simulink model "r2_ratty2_32k_r203"  (R2012b) (continued)
== system r2_ratty2_32k_r203/fft/fft_direct == (continued)
      mask prompts: n_inputs "number inputs"; biplex "Used in biplex FFT"; FFTSize "Size of FFT: (2^? pts)"; Coeffs "Coefficients: (0 to 2^(FFTSize-1)-1)"; StepPeriod "Coefficient Step Period: (2^?)"; coeff_bit_width "Coefficient Bit Width"; input_bit_width "Input Bit Width"; bin_pt_in "Input binary point"; async "asynchronous operation"; add_latency "Add Latency"; mult_latency "Mult Latency"; bram_latency "BRAM Latency"; conv_latency "Convert latency"; quantization "Quantization Behavior"; overflow "Overflow Behavior"; coeffs_bit_limit "Number bits above which to store stage's coefficients in BRAM (2^? bits)"; coeff_sharing "BRAM sharing in coeff storage"; coeff_decimation "Store a fraction of coeff factors where useful"; coeff_generation "Generate coeffs with multipliers where useful"; cal_bits "Number calibration locations when generating coeffs (2^?)"; n_bits_rotation "Feedback rotation vector resolution"; max_fanout "Maximum fanout"; use_hdl "Use behavioural HDL for multipliers"; use_embedded "Use embedded multipliers"; bitgrowth "Bit growth instead of shifting"; hardcode_shifts "Hardcode shift schedule"; dsp48_adders "Use DSP48s for adders"
      mask help: FFT internal block.
      mask init: same as r2_ratty2_32k_r203/fft/fft_direct/butterfly0_0
      settings: AttributesFormatString=twiddle_general
      mask TypeOptions quantization: Truncate | Round  (unbiased: +/- Inf) | Round  (unbiased: Even Values)
      mask TypeOptions overflow: Wrap | Saturate | Error
      mask Callback use_hdl:
      | % make mutually exclusive with use_embedded.
      | 
      | use_hdl = get_param(gcb, 'use_hdl');
      | if strcmp(use_hdl, 'on'),
      |     set_param_state(gcb, 'use_embedded', 'off');
      | else
      |     set_param_state(gcb, 'use_embedded', 'on');
      | end
      mask Callback use_embedded:
      | % make mutually exclusive with use_hdl.
      | 
      | use_hdl = get_param(gcb, 'use_embedded');
      | if strcmp(use_hdl, 'on'),
      |     set_param_state(gcb, 'use_hdl', 'off');
      | else
      |     set_param_state(gcb, 'use_hdl', 'on');
      | end
      mask Callback hardcode_shifts:
      | hardcode_shifts = get_param(gcb, 'hardcode_shifts');
      | set_param_state(gcb, 'downshift', hardcode_shifts);
      mask Callback dsp48_adders: %fft_callback_dsp48_adders(gcb);
  butterfly2_0 : Subsystem  AncestorBlock=casper_library_ffts/butterfly_direct  mask(butterfly_direct){n_inputs=1, biplex=off, FFTSize=16, Coeffs=[ 0 16384 8192 24576 4096 20480 12288 28672 2048 18432 10240 26624 6144 22528 14336 30720 1024 17408 9216 25600 5120 21504 13312 29696 3072 19456 11264 27648 7168 23552 15360 31744 512 16896 8704 25088 4608 20992 12800 29184 2560 18944 10752 27136 6656 23040 14848 31232 1536 17920 9728 26112 5632 22016 13824 30208 3584 19968 11776 28160 7680 24064 15872 32256 256 16640 8448 24832 4352 20736 12544 28928 2304 18688 10496 26880 6400 22784 14592 30976 1280 17664 9472 25856 5376 21760 13568 29952 3328 19712 11520 27904 7424 23808 15616 32000 768 17152 8960 25344 4864 21248 13056 29440 2816 19200 11008 27392 6912 23296 15104 31488 1792 18176 9984 26368 5888 22272 14080 30464 3840 20224 12032 28416 7936 24320 16128 32512 128 16512 8320 24704 4224 20608 12416 28800 2176 18560 10368 26752 6272 22656 14464 30848 1152 17536 9344 25728 5248 21632 13440 29824 3200 19584 11392 27776 7296 23680 15488 31872 640 17024 8832 25216 4736 21120 12928 29312 2688 19072 10880 27264 6784 23168 14976 31360 1664 18048 9856 26240 5760 22144 13952 30336 3712 20096 11904 28288 7808 24192 16000 32384 384 16768 8576 24960 4480 20864 12672 29056 2432 18816 10624 27008 6528 22912 14720 31104 1408 17792 9600 25984 5504 21888 13696 30080 3456 19840 11648 28032 7552 23936 15744 32128 896 17280 9088 25472 4992 21376 13184 29568 2944 19328 11136 27520 7040 23424 15232 31616 1920 18304 10112 26496 6016 22400 14208 30592 3968 20352 12160 28544 8064 24448 16256 32640 64 16448 8256 24640 4160 20544 12352 28736 2112 18496 10304 26688 6208 22592 14400 30784 1088 17472 9280 25664 5184 21568 13376 29760 3136 19520 11328 27712 7232 23616 15424 31808 576 16960 8768 25152 4672 21056 12864 29248 2624 19008 10816 27200 6720 23104 14912 31296 1600 17984 9792 26176 5696 22080 13888 30272 3648 20032 11840 28224 7744 24128 15936 32320 320 16704 8512 24896 4416 20800 12608 28992 2368 18752 10560 26944 6464 22848 14656 31040 1344 17728 9536 25920 5440 21824 13632 30016 3392 19776 11584 27968 7488 23872 15680 32064 832 17216 9024 25408 4928 21312 13120 29504 2880 19264 11072 27456 6976 23360 15168 31552 1856 18240 10048 26432 5952 22336 14144 30528 3904 20288 12096 28480 8000 24384 16192 32576 192 16576 8384 24768 4288 20672 12480 28864 2240 18624 10432 26816 6336 22720 14528 30912 1216 17600 9408 25792 5312 21696 13504 29888 3264 19648 11456 27840 7360 23744 15552 31936 704 17088 8896 25280 4800 21184 12992 29376 2752 19136 10944 27328 6848 23232 15040 31424 1728 18112 9920 26304 5824 22208 14016 30400 3776 20160 11968 28352 7872 24256 16064 32448 448 16832 8640 25024 4544 20928 12736 29120 2496 18880 10688 27072 6592 22976 14784 31168 1472 17856 9664 26048 5568 21952 13760 30144 3520 19904 11712 28096 7616 24000 15808 32192 960 17344 9152 25536 5056 21440 13248 29632 3008 19392 11200 27584 7104 23488 15296 31680 1984 18368 10176 26560 6080 22464 14272 30656 4032 20416 12224 28608 8128 24512 16320 32704 32 16416 8224 24608 4128 20512 12320 28704 2080 18464 10272 26656 6176 22560 14368 30752 1056 17440 9248 25632 5152 21536 13344 29728 3104 19488 11296 27680 7200 23584 15392 31776 544 16928 8736 25120 4640 21024 12832 29216 2592 18976 10784 27168 6688 23072 14880 31264 1568 17952 9760 26144 5664 22048 13856 30240 3616 20000 11808 28192 7712 24096 15904 32288 288 16672 8480 24864 4384 20768 12576 28960 2336 18720 10528 26912 6432 22816 14624 31008 1312 17696 9504 25888 5408 21792 13600 29984 3360 19744 11552 27936 7456 23840 15648 32032 800 17184 8992 25376 4896 21280 13088 29472 2848 19232 11040 27424 6944 23328 15136 31520 1824 18208 10016 26400 5920 22304 14112 30496 3872 20256 12064 28448 7968 24352 16160 32544 160 16544 8352 24736 4256 20640 12448 28832 2208 18592 10400 26784 6304 22688 14496 30880 1184 17568 9376 25760 5280 21664 13472 29856 3232 19616 11424 27808 7328 23712 15520 31904 672 17056 8864 25248 4768 21152 12960 29344 2720 19104 10912 27296 6816 23200 15008 31392 1696 18080 9888 26272 5792 22176 13984 30368 3744 20128 11936 28320 7840 24224 16032 32416 416 16800 8608 ...(+42377 chars)], StepPeriod=0, coeff_bit_width=18, input_bit_width=23, bin_pt_in=21, downshift=off, async=off, add_latency=4, mult_latency=5, bram_latency=12, conv_latency=5, quantization=Round  (unbiased: +/- Inf), overflow=Wrap, coeffs_bit_limit=10, coeff_sharing=off, coeff_decimation=off, coeff_generation=off, cal_bits=cal_bits, n_bits_rotation=n_bits_rotation, max_fanout=max_fanout, use_hdl=off, use_embedded=on, bitgrowth=off, hardcode_shifts=off, dsp48_adders=off}
      mask prompts: n_inputs "number inputs"; biplex "Used in biplex FFT"; FFTSize "Size of FFT: (2^? pts)"; Coeffs "Coefficients: (0 to 2^(FFTSize-1)-1)"; StepPeriod "Coefficient Step Period: (2^?)"; coeff_bit_width "Coefficient Bit Width"; input_bit_width "Input Bit Width"; bin_pt_in "Input binary point"; async "asynchronous operation"; add_latency "Add Latency"; mult_latency "Mult Latency"; bram_latency "BRAM Latency"; conv_latency "Convert latency"; quantization "Quantization Behavior"; overflow "Overflow Behavior"; coeffs_bit_limit "Number bits above which to store stage's coefficients in BRAM (2^? bits)"; coeff_sharing "BRAM sharing in coeff storage"; coeff_decimation "Store a fraction of coeff factors where useful"; coeff_generation "Generate coeffs with multipliers where useful"; cal_bits "Number calibration locations when generating coeffs (2^?)"; n_bits_rotation "Feedback rotation vector resolution"; max_fanout "Maximum fanout"; use_hdl "Use behavioural HDL for multipliers"; use_embedded "Use embedded multipliers"; bitgrowth "Bit growth instead of shifting"; hardcode_shifts "Hardcode shift schedule"; dsp48_adders "Use DSP48s for adders"
      mask help: FFT internal block.
      mask init: same as r2_ratty2_32k_r203/fft/fft_direct/butterfly0_0
      settings: AttributesFormatString=twiddle_general
      mask TypeOptions quantization: Truncate | Round  (unbiased: +/- Inf) | Round  (unbiased: Even Values)
      mask TypeOptions overflow: Wrap | Saturate | Error
      mask Callback use_hdl:
      | % make mutually exclusive with use_embedded.
      | 
      | use_hdl = get_param(gcb, 'use_hdl');
      | if strcmp(use_hdl, 'on'),
      |     set_param_state(gcb, 'use_embedded', 'off');
      | else
      |     set_param_state(gcb, 'use_embedded', 'on');
      | end
      mask Callback use_embedded:
      | % make mutually exclusive with use_hdl.
      | 
      | use_hdl = get_param(gcb, 'use_embedded');
      | if strcmp(use_hdl, 'on'),
      |     set_param_state(gcb, 'use_hdl', 'off');
      | else
      |     set_param_state(gcb, 'use_hdl', 'on');
      | end
      mask Callback hardcode_shifts:
      | hardcode_shifts = get_param(gcb, 'hardcode_shifts');
      | set_param_state(gcb, 'downshift', hardcode_shifts);
      mask Callback dsp48_adders: %fft_callback_dsp48_adders(gcb);
  butterfly2_1 : Subsystem  AncestorBlock=casper_library_ffts/butterfly_direct  mask(butterfly_direct){n_inputs=1, biplex=off, FFTSize=16, Coeffs=[ 1 16385 8193 24577 4097 20481 12289 28673 2049 18433 10241 26625 6145 22529 14337 30721 1025 17409 9217 25601 5121 21505 13313 29697 3073 19457 11265 27649 7169 23553 15361 31745 513 16897 8705 25089 4609 20993 12801 29185 2561 18945 10753 27137 6657 23041 14849 31233 1537 17921 9729 26113 5633 22017 13825 30209 3585 19969 11777 28161 7681 24065 15873 32257 257 16641 8449 24833 4353 20737 12545 28929 2305 18689 10497 26881 6401 22785 14593 30977 1281 17665 9473 25857 5377 21761 13569 29953 3329 19713 11521 27905 7425 23809 15617 32001 769 17153 8961 25345 4865 21249 13057 29441 2817 19201 11009 27393 6913 23297 15105 31489 1793 18177 9985 26369 5889 22273 14081 30465 3841 20225 12033 28417 7937 24321 16129 32513 129 16513 8321 24705 4225 20609 12417 28801 2177 18561 10369 26753 6273 22657 14465 30849 1153 17537 9345 25729 5249 21633 13441 29825 3201 19585 11393 27777 7297 23681 15489 31873 641 17025 8833 25217 4737 21121 12929 29313 2689 19073 10881 27265 6785 23169 14977 31361 1665 18049 9857 26241 5761 22145 13953 30337 3713 20097 11905 28289 7809 24193 16001 32385 385 16769 8577 24961 4481 20865 12673 29057 2433 18817 10625 27009 6529 22913 14721 31105 1409 17793 9601 25985 5505 21889 13697 30081 3457 19841 11649 28033 7553 23937 15745 32129 897 17281 9089 25473 4993 21377 13185 29569 2945 19329 11137 27521 7041 23425 15233 31617 1921 18305 10113 26497 6017 22401 14209 30593 3969 20353 12161 28545 8065 24449 16257 32641 65 16449 8257 24641 4161 20545 12353 28737 2113 18497 10305 26689 6209 22593 14401 30785 1089 17473 9281 25665 5185 21569 13377 29761 3137 19521 11329 27713 7233 23617 15425 31809 577 16961 8769 25153 4673 21057 12865 29249 2625 19009 10817 27201 6721 23105 14913 31297 1601 17985 9793 26177 5697 22081 13889 30273 3649 20033 11841 28225 7745 24129 15937 32321 321 16705 8513 24897 4417 20801 12609 28993 2369 18753 10561 26945 6465 22849 14657 31041 1345 17729 9537 25921 5441 21825 13633 30017 3393 19777 11585 27969 7489 23873 15681 32065 833 17217 9025 25409 4929 21313 13121 29505 2881 19265 11073 27457 6977 23361 15169 31553 1857 18241 10049 26433 5953 22337 14145 30529 3905 20289 12097 28481 8001 24385 16193 32577 193 16577 8385 24769 4289 20673 12481 28865 2241 18625 10433 26817 6337 22721 14529 30913 1217 17601 9409 25793 5313 21697 13505 29889 3265 19649 11457 27841 7361 23745 15553 31937 705 17089 8897 25281 4801 21185 12993 29377 2753 19137 10945 27329 6849 23233 15041 31425 1729 18113 9921 26305 5825 22209 14017 30401 3777 20161 11969 28353 7873 24257 16065 32449 449 16833 8641 25025 4545 20929 12737 29121 2497 18881 10689 27073 6593 22977 14785 31169 1473 17857 9665 26049 5569 21953 13761 30145 3521 19905 11713 28097 7617 24001 15809 32193 961 17345 9153 25537 5057 21441 13249 29633 3009 19393 11201 27585 7105 23489 15297 31681 1985 18369 10177 26561 6081 22465 14273 30657 4033 20417 12225 28609 8129 24513 16321 32705 33 16417 8225 24609 4129 20513 12321 28705 2081 18465 10273 26657 6177 22561 14369 30753 1057 17441 9249 25633 5153 21537 13345 29729 3105 19489 11297 27681 7201 23585 15393 31777 545 16929 8737 25121 4641 21025 12833 29217 2593 18977 10785 27169 6689 23073 14881 31265 1569 17953 9761 26145 5665 22049 13857 30241 3617 20001 11809 28193 7713 24097 15905 32289 289 16673 8481 24865 4385 20769 12577 28961 2337 18721 10529 26913 6433 22817 14625 31009 1313 17697 9505 25889 5409 21793 13601 29985 3361 19745 11553 27937 7457 23841 15649 32033 801 17185 8993 25377 4897 21281 13089 29473 2849 19233 11041 27425 6945 23329 15137 31521 1825 18209 10017 26401 5921 22305 14113 30497 3873 20257 12065 28449 7969 24353 16161 32545 161 16545 8353 24737 4257 20641 12449 28833 2209 18593 10401 26785 6305 22689 14497 30881 1185 17569 9377 25761 5281 21665 13473 29857 3233 19617 11425 27809 7329 23713 15521 31905 673 17057 8865 25249 4769 21153 12961 29345 2721 19105 10913 27297 6817 23201 15009 31393 1697 18081 9889 26273 5793 22177 13985 30369 3745 20129 11937 28321 7841 24225 16033 32417 417 16801 8609 ...(+42377 chars)], StepPeriod=0, coeff_bit_width=18, input_bit_width=23, bin_pt_in=21, downshift=off, async=off, add_latency=4, mult_latency=5, bram_latency=12, conv_latency=5, quantization=Round  (unbiased: +/- Inf), overflow=Wrap, coeffs_bit_limit=10, coeff_sharing=off, coeff_decimation=off, coeff_generation=off, cal_bits=cal_bits, n_bits_rotation=n_bits_rotation, max_fanout=max_fanout, use_hdl=off, use_embedded=on, bitgrowth=off, hardcode_shifts=off, dsp48_adders=off}
      mask prompts: n_inputs "number inputs"; biplex "Used in biplex FFT"; FFTSize "Size of FFT: (2^? pts)"; Coeffs "Coefficients: (0 to 2^(FFTSize-1)-1)"; StepPeriod "Coefficient Step Period: (2^?)"; coeff_bit_width "Coefficient Bit Width"; input_bit_width "Input Bit Width"; bin_pt_in "Input binary point"; async "asynchronous operation"; add_latency "Add Latency"; mult_latency "Mult Latency"; bram_latency "BRAM Latency"; conv_latency "Convert latency"; quantization "Quantization Behavior"; overflow "Overflow Behavior"; coeffs_bit_limit "Number bits above which to store stage's coefficients in BRAM (2^? bits)"; coeff_sharing "BRAM sharing in coeff storage"; coeff_decimation "Store a fraction of coeff factors where useful"; coeff_generation "Generate coeffs with multipliers where useful"; cal_bits "Number calibration locations when generating coeffs (2^?)"; n_bits_rotation "Feedback rotation vector resolution"; max_fanout "Maximum fanout"; use_hdl "Use behavioural HDL for multipliers"; use_embedded "Use embedded multipliers"; bitgrowth "Bit growth instead of shifting"; hardcode_shifts "Hardcode shift schedule"; dsp48_adders "Use DSP48s for adders"
      mask help: FFT internal block.
      mask init: same as r2_ratty2_32k_r203/fft/fft_direct/butterfly0_0
      settings: AttributesFormatString=twiddle_general
      mask TypeOptions quantization: Truncate | Round  (unbiased: +/- Inf) | Round  (unbiased: Even Values)
      mask TypeOptions overflow: Wrap | Saturate | Error
      mask Callback use_hdl:
      | % make mutually exclusive with use_embedded.
      | 
      | use_hdl = get_param(gcb, 'use_hdl');
      | if strcmp(use_hdl, 'on'),
      |     set_param_state(gcb, 'use_embedded', 'off');
      | else
      |     set_param_state(gcb, 'use_embedded', 'on');
      | end
      mask Callback use_embedded:
      | % make mutually exclusive with use_hdl.
      | 
      | use_hdl = get_param(gcb, 'use_embedded');
      | if strcmp(use_hdl, 'on'),
      |     set_param_state(gcb, 'use_hdl', 'off');
      | else
      |     set_param_state(gcb, 'use_hdl', 'on');
      | end
      mask Callback hardcode_shifts:
      | hardcode_shifts = get_param(gcb, 'hardcode_shifts');
      | set_param_state(gcb, 'downshift', hardcode_shifts);
      mask Callback dsp48_adders: %fft_callback_dsp48_adders(gcb);
  butterfly2_2 : Subsystem  AncestorBlock=casper_library_ffts/butterfly_direct  mask(butterfly_direct){n_inputs=1, biplex=off, FFTSize=16, Coeffs=[ 2 16386 8194 24578 4098 20482 12290 28674 2050 18434 10242 26626 6146 22530 14338 30722 1026 17410 9218 25602 5122 21506 13314 29698 3074 19458 11266 27650 7170 23554 15362 31746 514 16898 8706 25090 4610 20994 12802 29186 2562 18946 10754 27138 6658 23042 14850 31234 1538 17922 9730 26114 5634 22018 13826 30210 3586 19970 11778 28162 7682 24066 15874 32258 258 16642 8450 24834 4354 20738 12546 28930 2306 18690 10498 26882 6402 22786 14594 30978 1282 17666 9474 25858 5378 21762 13570 29954 3330 19714 11522 27906 7426 23810 15618 32002 770 17154 8962 25346 4866 21250 13058 29442 2818 19202 11010 27394 6914 23298 15106 31490 1794 18178 9986 26370 5890 22274 14082 30466 3842 20226 12034 28418 7938 24322 16130 32514 130 16514 8322 24706 4226 20610 12418 28802 2178 18562 10370 26754 6274 22658 14466 30850 1154 17538 9346 25730 5250 21634 13442 29826 3202 19586 11394 27778 7298 23682 15490 31874 642 17026 8834 25218 4738 21122 12930 29314 2690 19074 10882 27266 6786 23170 14978 31362 1666 18050 9858 26242 5762 22146 13954 30338 3714 20098 11906 28290 7810 24194 16002 32386 386 16770 8578 24962 4482 20866 12674 29058 2434 18818 10626 27010 6530 22914 14722 31106 1410 17794 9602 25986 5506 21890 13698 30082 3458 19842 11650 28034 7554 23938 15746 32130 898 17282 9090 25474 4994 21378 13186 29570 2946 19330 11138 27522 7042 23426 15234 31618 1922 18306 10114 26498 6018 22402 14210 30594 3970 20354 12162 28546 8066 24450 16258 32642 66 16450 8258 24642 4162 20546 12354 28738 2114 18498 10306 26690 6210 22594 14402 30786 1090 17474 9282 25666 5186 21570 13378 29762 3138 19522 11330 27714 7234 23618 15426 31810 578 16962 8770 25154 4674 21058 12866 29250 2626 19010 10818 27202 6722 23106 14914 31298 1602 17986 9794 26178 5698 22082 13890 30274 3650 20034 11842 28226 7746 24130 15938 32322 322 16706 8514 24898 4418 20802 12610 28994 2370 18754 10562 26946 6466 22850 14658 31042 1346 17730 9538 25922 5442 21826 13634 30018 3394 19778 11586 27970 7490 23874 15682 32066 834 17218 9026 25410 4930 21314 13122 29506 2882 19266 11074 27458 6978 23362 15170 31554 1858 18242 10050 26434 5954 22338 14146 30530 3906 20290 12098 28482 8002 24386 16194 32578 194 16578 8386 24770 4290 20674 12482 28866 2242 18626 10434 26818 6338 22722 14530 30914 1218 17602 9410 25794 5314 21698 13506 29890 3266 19650 11458 27842 7362 23746 15554 31938 706 17090 8898 25282 4802 21186 12994 29378 2754 19138 10946 27330 6850 23234 15042 31426 1730 18114 9922 26306 5826 22210 14018 30402 3778 20162 11970 28354 7874 24258 16066 32450 450 16834 8642 25026 4546 20930 12738 29122 2498 18882 10690 27074 6594 22978 14786 31170 1474 17858 9666 26050 5570 21954 13762 30146 3522 19906 11714 28098 7618 24002 15810 32194 962 17346 9154 25538 5058 21442 13250 29634 3010 19394 11202 27586 7106 23490 15298 31682 1986 18370 10178 26562 6082 22466 14274 30658 4034 20418 12226 28610 8130 24514 16322 32706 34 16418 8226 24610 4130 20514 12322 28706 2082 18466 10274 26658 6178 22562 14370 30754 1058 17442 9250 25634 5154 21538 13346 29730 3106 19490 11298 27682 7202 23586 15394 31778 546 16930 8738 25122 4642 21026 12834 29218 2594 18978 10786 27170 6690 23074 14882 31266 1570 17954 9762 26146 5666 22050 13858 30242 3618 20002 11810 28194 7714 24098 15906 32290 290 16674 8482 24866 4386 20770 12578 28962 2338 18722 10530 26914 6434 22818 14626 31010 1314 17698 9506 25890 5410 21794 13602 29986 3362 19746 11554 27938 7458 23842 15650 32034 802 17186 8994 25378 4898 21282 13090 29474 2850 19234 11042 27426 6946 23330 15138 31522 1826 18210 10018 26402 5922 22306 14114 30498 3874 20258 12066 28450 7970 24354 16162 32546 162 16546 8354 24738 4258 20642 12450 28834 2210 18594 10402 26786 6306 22690 14498 30882 1186 17570 9378 25762 5282 21666 13474 29858 3234 19618 11426 27810 7330 23714 15522 31906 674 17058 8866 25250 4770 21154 12962 29346 2722 19106 10914 27298 6818 23202 15010 31394 1698 18082 9890 26274 5794 22178 13986 30370 3746 20130 11938 28322 7842 24226 16034 32418 418 16802 8610 ...(+42378 chars)], StepPeriod=0, coeff_bit_width=18, input_bit_width=23, bin_pt_in=21, downshift=off, async=off, add_latency=4, mult_latency=5, bram_latency=12, conv_latency=5, quantization=Round  (unbiased: +/- Inf), overflow=Wrap, coeffs_bit_limit=10, coeff_sharing=off, coeff_decimation=off, coeff_generation=off, cal_bits=cal_bits, n_bits_rotation=n_bits_rotation, max_fanout=max_fanout, use_hdl=off, use_embedded=on, bitgrowth=off, hardcode_shifts=off, dsp48_adders=off}
      mask prompts: n_inputs "number inputs"; biplex "Used in biplex FFT"; FFTSize "Size of FFT: (2^? pts)"; Coeffs "Coefficients: (0 to 2^(FFTSize-1)-1)"; StepPeriod "Coefficient Step Period: (2^?)"; coeff_bit_width "Coefficient Bit Width"; input_bit_width "Input Bit Width"; bin_pt_in "Input binary point"; async "asynchronous operation"; add_latency "Add Latency"; mult_latency "Mult Latency"; bram_latency "BRAM Latency"; conv_latency "Convert latency"; quantization "Quantization Behavior"; overflow "Overflow Behavior"; coeffs_bit_limit "Number bits above which to store stage's coefficients in BRAM (2^? bits)"; coeff_sharing "BRAM sharing in coeff storage"; coeff_decimation "Store a fraction of coeff factors where useful"; coeff_generation "Generate coeffs with multipliers where useful"; cal_bits "Number calibration locations when generating coeffs (2^?)"; n_bits_rotation "Feedback rotation vector resolution"; max_fanout "Maximum fanout"; use_hdl "Use behavioural HDL for multipliers"; use_embedded "Use embedded multipliers"; bitgrowth "Bit growth instead of shifting"; hardcode_shifts "Hardcode shift schedule"; dsp48_adders "Use DSP48s for adders"
      mask help: FFT internal block.
      mask init: same as r2_ratty2_32k_r203/fft/fft_direct/butterfly0_0
      settings: AttributesFormatString=twiddle_general
      mask TypeOptions quantization: Truncate | Round  (unbiased: +/- Inf) | Round  (unbiased: Even Values)
      mask TypeOptions overflow: Wrap | Saturate | Error
      mask Callback use_hdl:
      | % make mutually exclusive with use_embedded.
      | 
      | use_hdl = get_param(gcb, 'use_hdl');
      | if strcmp(use_hdl, 'on'),
      |     set_param_state(gcb, 'use_embedded', 'off');
      | else
      |     set_param_state(gcb, 'use_embedded', 'on');
      | end
      mask Callback use_embedded:
      | % make mutually exclusive with use_hdl.
      | 
      | use_hdl = get_param(gcb, 'use_embedded');
      | if strcmp(use_hdl, 'on'),
      |     set_param_state(gcb, 'use_hdl', 'off');
      | else
      |     set_param_state(gcb, 'use_hdl', 'on');
      | end
      mask Callback hardcode_shifts:
      | hardcode_shifts = get_param(gcb, 'hardcode_shifts');
      | set_param_state(gcb, 'downshift', hardcode_shifts);
      mask Callback dsp48_adders: %fft_callback_dsp48_adders(gcb);
  butterfly2_3 : Subsystem  AncestorBlock=casper_library_ffts/butterfly_direct  mask(butterfly_direct){n_inputs=1, biplex=off, FFTSize=16, Coeffs=[ 3 16387 8195 24579 4099 20483 12291 28675 2051 18435 10243 26627 6147 22531 14339 30723 1027 17411 9219 25603 5123 21507 13315 29699 3075 19459 11267 27651 7171 23555 15363 31747 515 16899 8707 25091 4611 20995 12803 29187 2563 18947 10755 27139 6659 23043 14851 31235 1539 17923 9731 26115 5635 22019 13827 30211 3587 19971 11779 28163 7683 24067 15875 32259 259 16643 8451 24835 4355 20739 12547 28931 2307 18691 10499 26883 6403 22787 14595 30979 1283 17667 9475 25859 5379 21763 13571 29955 3331 19715 11523 27907 7427 23811 15619 32003 771 17155 8963 25347 4867 21251 13059 29443 2819 19203 11011 27395 6915 23299 15107 31491 1795 18179 9987 26371 5891 22275 14083 30467 3843 20227 12035 28419 7939 24323 16131 32515 131 16515 8323 24707 4227 20611 12419 28803 2179 18563 10371 26755 6275 22659 14467 30851 1155 17539 9347 25731 5251 21635 13443 29827 3203 19587 11395 27779 7299 23683 15491 31875 643 17027 8835 25219 4739 21123 12931 29315 2691 19075 10883 27267 6787 23171 14979 31363 1667 18051 9859 26243 5763 22147 13955 30339 3715 20099 11907 28291 7811 24195 16003 32387 387 16771 8579 24963 4483 20867 12675 29059 2435 18819 10627 27011 6531 22915 14723 31107 1411 17795 9603 25987 5507 21891 13699 30083 3459 19843 11651 28035 7555 23939 15747 32131 899 17283 9091 25475 4995 21379 13187 29571 2947 19331 11139 27523 7043 23427 15235 31619 1923 18307 10115 26499 6019 22403 14211 30595 3971 20355 12163 28547 8067 24451 16259 32643 67 16451 8259 24643 4163 20547 12355 28739 2115 18499 10307 26691 6211 22595 14403 30787 1091 17475 9283 25667 5187 21571 13379 29763 3139 19523 11331 27715 7235 23619 15427 31811 579 16963 8771 25155 4675 21059 12867 29251 2627 19011 10819 27203 6723 23107 14915 31299 1603 17987 9795 26179 5699 22083 13891 30275 3651 20035 11843 28227 7747 24131 15939 32323 323 16707 8515 24899 4419 20803 12611 28995 2371 18755 10563 26947 6467 22851 14659 31043 1347 17731 9539 25923 5443 21827 13635 30019 3395 19779 11587 27971 7491 23875 15683 32067 835 17219 9027 25411 4931 21315 13123 29507 2883 19267 11075 27459 6979 23363 15171 31555 1859 18243 10051 26435 5955 22339 14147 30531 3907 20291 12099 28483 8003 24387 16195 32579 195 16579 8387 24771 4291 20675 12483 28867 2243 18627 10435 26819 6339 22723 14531 30915 1219 17603 9411 25795 5315 21699 13507 29891 3267 19651 11459 27843 7363 23747 15555 31939 707 17091 8899 25283 4803 21187 12995 29379 2755 19139 10947 27331 6851 23235 15043 31427 1731 18115 9923 26307 5827 22211 14019 30403 3779 20163 11971 28355 7875 24259 16067 32451 451 16835 8643 25027 4547 20931 12739 29123 2499 18883 10691 27075 6595 22979 14787 31171 1475 17859 9667 26051 5571 21955 13763 30147 3523 19907 11715 28099 7619 24003 15811 32195 963 17347 9155 25539 5059 21443 13251 29635 3011 19395 11203 27587 7107 23491 15299 31683 1987 18371 10179 26563 6083 22467 14275 30659 4035 20419 12227 28611 8131 24515 16323 32707 35 16419 8227 24611 4131 20515 12323 28707 2083 18467 10275 26659 6179 22563 14371 30755 1059 17443 9251 25635 5155 21539 13347 29731 3107 19491 11299 27683 7203 23587 15395 31779 547 16931 8739 25123 4643 21027 12835 29219 2595 18979 10787 27171 6691 23075 14883 31267 1571 17955 9763 26147 5667 22051 13859 30243 3619 20003 11811 28195 7715 24099 15907 32291 291 16675 8483 24867 4387 20771 12579 28963 2339 18723 10531 26915 6435 22819 14627 31011 1315 17699 9507 25891 5411 21795 13603 29987 3363 19747 11555 27939 7459 23843 15651 32035 803 17187 8995 25379 4899 21283 13091 29475 2851 19235 11043 27427 6947 23331 15139 31523 1827 18211 10019 26403 5923 22307 14115 30499 3875 20259 12067 28451 7971 24355 16163 32547 163 16547 8355 24739 4259 20643 12451 28835 2211 18595 10403 26787 6307 22691 14499 30883 1187 17571 9379 25763 5283 21667 13475 29859 3235 19619 11427 27811 7331 23715 15523 31907 675 17059 8867 25251 4771 21155 12963 29347 2723 19107 10915 27299 6819 23203 15011 31395 1699 18083 9891 26275 5795 22179 13987 30371 3747 20131 11939 28323 7843 24227 16035 32419 419 16803 8611 ...(+42378 chars)], StepPeriod=0, coeff_bit_width=18, input_bit_width=23, bin_pt_in=21, downshift=off, async=off, add_latency=4, mult_latency=5, bram_latency=12, conv_latency=5, quantization=Round  (unbiased: +/- Inf), overflow=Wrap, coeffs_bit_limit=10, coeff_sharing=off, coeff_decimation=off, coeff_generation=off, cal_bits=cal_bits, n_bits_rotation=n_bits_rotation, max_fanout=max_fanout, use_hdl=off, use_embedded=on, bitgrowth=off, hardcode_shifts=off, dsp48_adders=off}
      mask prompts: n_inputs "number inputs"; biplex "Used in biplex FFT"; FFTSize "Size of FFT: (2^? pts)"; Coeffs "Coefficients: (0 to 2^(FFTSize-1)-1)"; StepPeriod "Coefficient Step Period: (2^?)"; coeff_bit_width "Coefficient Bit Width"; input_bit_width "Input Bit Width"; bin_pt_in "Input binary point"; async "asynchronous operation"; add_latency "Add Latency"; mult_latency "Mult Latency"; bram_latency "BRAM Latency"; conv_latency "Convert latency"; quantization "Quantization Behavior"; overflow "Overflow Behavior"; coeffs_bit_limit "Number bits above which to store stage's coefficients in BRAM (2^? bits)"; coeff_sharing "BRAM sharing in coeff storage"; coeff_decimation "Store a fraction of coeff factors where useful"; coeff_generation "Generate coeffs with multipliers where useful"; cal_bits "Number calibration locations when generating coeffs (2^?)"; n_bits_rotation "Feedback rotation vector resolution"; max_fanout "Maximum fanout"; use_hdl "Use behavioural HDL for multipliers"; use_embedded "Use embedded multipliers"; bitgrowth "Bit growth instead of shifting"; hardcode_shifts "Hardcode shift schedule"; dsp48_adders "Use DSP48s for adders"
      mask help: FFT internal block.
      mask init: same as r2_ratty2_32k_r203/fft/fft_direct/butterfly0_0
      settings: AttributesFormatString=twiddle_general
      mask TypeOptions quantization: Truncate | Round  (unbiased: +/- Inf) | Round  (unbiased: Even Values)
      mask TypeOptions overflow: Wrap | Saturate | Error
      mask Callback use_hdl:
      | % make mutually exclusive with use_embedded.
      | 
      | use_hdl = get_param(gcb, 'use_hdl');
      | if strcmp(use_hdl, 'on'),
      |     set_param_state(gcb, 'use_embedded', 'off');
      | else
      |     set_param_state(gcb, 'use_embedded', 'on');
      | end
      mask Callback use_embedded:
      | % make mutually exclusive with use_hdl.
      | 
      | use_hdl = get_param(gcb, 'use_embedded');
      | if strcmp(use_hdl, 'on'),
      |     set_param_state(gcb, 'use_hdl', 'off');
      | else
      |     set_param_state(gcb, 'use_hdl', 'on');
      | end
      mask Callback hardcode_shifts:
      | hardcode_shifts = get_param(gcb, 'hardcode_shifts');
      | set_param_state(gcb, 'downshift', hardcode_shifts);
      mask Callback dsp48_adders: %fft_callback_dsp48_adders(gcb);
  combine : xbsIndex_r4/Logical  logical_function=OR  inputs=4  en=off  latency=1  precision=Full  arith_type=Unsigned  n_bits=16  bin_pt=0  align_bp=on  dbl_ovrd=off  block_type=logical
  of_0 : xbsIndex_r4/Delay  rst=off  infoeditControl=Selection of Reset will increase slice count due to use of real FFs and instead of SRLs  en=off  latency=0  dbl_ovrd=off  reg_retiming=off  block_type=delay
  of_1 : xbsIndex_r4/Concat  num_inputs=2  dbl_ovrd=off  block_type=concat
  of_2 : xbsIndex_r4/Concat  num_inputs=4  dbl_ovrd=off  block_type=concat
  of_expand : Subsystem  AncestorBlock=casper_library_flow_control/bus_expand  mask(bus_expand){mode=divisions of equal size, outputNum=4, outputWidth=1, outputBinaryPt=0, outputArithmeticType=0, show_format=off, outputToWorkspace=off, variablePrefix=out, outputToModelAsWell=on}
      mask prompts: outputNum "Number of outputs:"; outputWidth "Output width:"; outputBinaryPt "Output binary point position:"; outputArithmeticType "Output arithmetic type (ufix=0, fix=1, bool=2):"; show_format "Print format string?"; outputToWorkspace "Output to workspace?"; variablePrefix "Variable name prefix:"; outputToModelAsWell "Output to model as well?"
      mask help:
      | Expands a 'bus' made using a bus_create or Xilinx
      | concat block.
      | The input will be divided into the specified number
      | of outputs with the specified arithmetic types.
      | Divisions are made from most significant bit.
      mask init: same as r2_ratty2_32k_r203/fft/fft_biplex_real_4x/pol0_debus
      settings: AttributesFormatString=4 outputs
      mask TypeOptions mode: divisions of equal size | divisions of arbitrary size
      mask Callback mode: bus_expand_callback();
      mask Callback outputWidth: bus_expand_callback();
      mask Callback outputToWorkspace: bus_expand_callback();
  of_or : xbsIndex_r4/Logical  logical_function=OR  inputs=3  en=off  latency=1  precision=Full  arith_type=Unsigned  n_bits=16  bin_pt=0  align_bp=on  dbl_ovrd=off  block_type=logical
  slice0 : xbsIndex_r4/Slice  nbits=1  boolean_output=on  mode=Lower Bit Location + Width  bit1=0  base1=MSB of Input  bit0=0  base0=LSB of Input  dbl_ovrd=off  block_type=slice
  slice1 : xbsIndex_r4/Slice  nbits=1  boolean_output=on  mode=Lower Bit Location + Width  bit1=0  base1=MSB of Input  bit0=1  base0=LSB of Input  dbl_ovrd=off  block_type=slice
  slice2 : xbsIndex_r4/Slice  nbits=1  boolean_output=on  mode=Lower Bit Location + Width  bit1=0  base1=MSB of Input  bit0=2  base0=LSB of Input  dbl_ovrd=off  block_type=slice
  synct1 : Terminator
  synct2 : Terminator
  synct3 : Terminator
  sync_out : Outport
  out00 : Outport  Port=2
  out01 : Outport  Port=3
  out02 : Outport  Port=4
  out03 : Outport  Port=5
  out04 : Outport  Port=6
  out05 : Outport  Port=7
  out06 : Outport  Port=8
  out07 : Outport  Port=9
  of : Outport  Port=10
  wires:
  in00/1 -> abus/1(in1)
  adebus2_0/1(msb_lsb_out1) -> out00/1
  in01/1 -> abus/2(in2)
  adebus2_2/1(msb_lsb_out1) -> out01/1
  in02/1 -> abus/3(in3)
  adebus2_1/1(msb_lsb_out1) -> out02/1
  in03/1 -> abus/4(in4)
  adebus2_3/1(msb_lsb_out1) -> out03/1
  in04/1 -> bbus/1(in1)
  bdebus2_0/1(msb_lsb_out1) -> out04/1
  in05/1 -> bbus/2(in2)
  bdebus2_2/1(msb_lsb_out1) -> out05/1
  in06/1 -> bbus/3(in3)
  bdebus2_1/1(msb_lsb_out1) -> out06/1
  in07/1 -> bbus/4(in4)
  bdebus2_3/1(msb_lsb_out1) -> out07/1
  of_or/1 -> of_expand/1(bus_in)
  of_expand/1(msb_out4) -> combine/1
  of_expand/2(out3) -> combine/2
  of_expand/3(out2) -> combine/3
  of_expand/4(lsb_out1) -> combine/4
  combine/1 -> of/1
  shift/1 -> slice0/1, slice1/1, slice2/1
  of_0/1 -> of_or/1
  sync/1 -> butterfly0_0/3(sync_in)
  slice0/1 -> butterfly0_0/4(shift)
  abus/1(bus_out) -> butterfly0_0/1(a)
  bbus/1(bus_out) -> butterfly0_0/2(b)
  butterfly0_0/3(of) -> of_0/1
  butterfly0_0/1(a+bw) -> adebus0_0/1(bus_in)
  butterfly0_0/2(a-bw) -> bdebus0_0/1(bus_in)
  of_1/1 -> of_or/2
  butterfly0_0/4(sync_out) -> butterfly1_0/3(sync_in), butterfly1_1/3(sync_in)
  slice1/1 -> butterfly1_0/4(shift), butterfly1_1/4(shift)
  adebus0_0/1(msb_out2) -> butterfly1_0/1(a)
  adebus0_0/2(lsb_out1) -> butterfly1_0/2(b)
  butterfly1_0/3(of) -> of_1/1
  butterfly1_0/1(a+bw) -> adebus1_0/1(bus_in)
  butterfly1_0/2(a-bw) -> bdebus1_0/1(bus_in)
  bdebus0_0/1(msb_out2) -> butterfly1_1/1(a)
  bdebus0_0/2(lsb_out1) -> butterfly1_1/2(b)
  butterfly1_1/3(of) -> of_1/2
  butterfly1_1/1(a+bw) -> adebus1_1/1(bus_in)
  butterfly1_1/2(a-bw) -> bdebus1_1/1(bus_in)
  of_2/1 -> of_or/3
  butterfly1_0/4(sync_out) -> butterfly2_0/3(sync_in), butterfly2_1/3(sync_in)
  butterfly2_0/4(sync_out) -> sync_out/1
  slice2/1 -> butterfly2_0/4(shift), butterfly2_1/4(shift), butterfly2_2/4(shift), butterfly2_3/4(shift)
  adebus1_0/1(msb_out2) -> butterfly2_0/1(a)
  adebus1_0/2(lsb_out1) -> butterfly2_0/2(b)
  butterfly2_0/3(of) -> of_2/1
  butterfly2_1/4(sync_out) -> synct1/1
  bdebus1_0/1(msb_out2) -> butterfly2_1/1(a)
  bdebus1_0/2(lsb_out1) -> butterfly2_1/2(b)
  butterfly2_1/3(of) -> of_2/2
  butterfly1_1/4(sync_out) -> butterfly2_2/3(sync_in), butterfly2_3/3(sync_in)
  butterfly2_2/4(sync_out) -> synct2/1
  adebus1_1/1(msb_out2) -> butterfly2_2/1(a)
  adebus1_1/2(lsb_out1) -> butterfly2_2/2(b)
  butterfly2_2/3(of) -> of_2/3
  butterfly2_3/4(sync_out) -> synct3/1
  bdebus1_1/1(msb_out2) -> butterfly2_3/1(a)
  bdebus1_1/2(lsb_out1) -> butterfly2_3/2(b)
  butterfly2_3/3(of) -> of_2/4
  butterfly2_0/1(a+bw) -> adebus2_0/1(bus_in)
  butterfly2_0/2(a-bw) -> bdebus2_0/1(bus_in)
  butterfly2_1/1(a+bw) -> adebus2_1/1(bus_in)
  butterfly2_1/2(a-bw) -> bdebus2_1/1(bus_in)
  butterfly2_2/1(a+bw) -> adebus2_2/1(bus_in)
  butterfly2_2/2(a-bw) -> bdebus2_2/1(bus_in)
  butterfly2_3/1(a+bw) -> adebus2_3/1(bus_in)
  butterfly2_3/2(a-bw) -> bdebus2_3/1(bus_in)
